simulink model "PlanarModelandControl"  (R2026a) (continued)
== system PlanarModelandControl/Planta/Dinamica do Veículo/Pacejka/Pacejka Long RR == (continued)
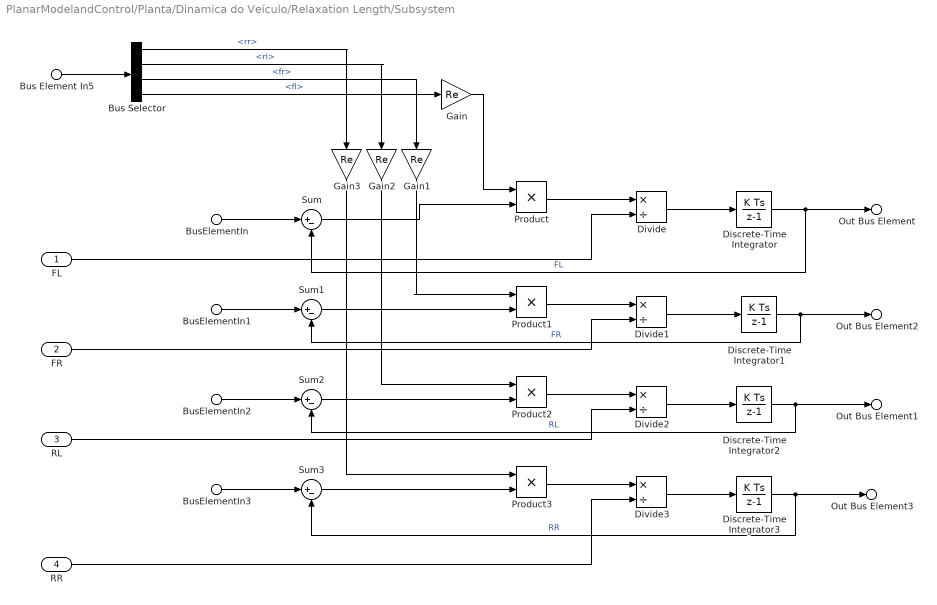
== system PlanarModelandControl/Planta/Dinamica do Veículo/Relaxation Length/Subsystem ==
  FL : Inport
  FR : Inport  Port=2
  RL : Inport  Port=3
  RR : Inport  Port=4
  BusElementIn : Inport  Port=5  PortName=SlipAnges  Element=SA_FL
  BusElementIn1 : Inport  Port=5  PortName=SlipAnges  Element=SA_FR
  BusElementIn2 : Inport  Port=5  PortName=SlipAnges  Element=SA_RL
  BusElementIn3 : Inport  Port=5  PortName=SlipAnges  Element=SA_RR
  Bus Element In5 : Inport  Port=6  PortName=omega
  Bus Selector : BusSelector  OutputSignals=rr,rl,fr,fl
  Discrete-Time Integrator{,1..3} (4 alike) : DiscreteIntegrator  SampleTime=-1  ICPrevOutput=DiscIntNeverNeededParam  ICPrevScaledInput=DiscIntNeverNeededParam
  Divide{,1..3} (4 alike) : Product  Inputs=*/
  Gain{,1..3} (4 alike) : Gain  Gain=Re
  Product{,1..3} (4 alike) : Product
  Sum{,1..3} (4 alike) : Sum  Inputs=|+-
  Out Bus Element : Outport  PortName=RelaxionLength  Element=SAs_FL
  Out Bus Element3 : Outport  PortName=RelaxionLength  Element=SAs_RR
  Out Bus Element2 : Outport  PortName=RelaxionLength  Element=SAs_FR
  Out Bus Element1 : Outport  PortName=RelaxionLength  Element=SAs_RL
  wires:
  BusElementIn/1 -> Sum/1
  BusElementIn1/1 -> Sum1/1
  BusElementIn2/1 -> Sum2/1
  BusElementIn3/1 -> Sum3/1
  Bus Element In5/1 -> Bus Selector/1
  Bus Selector/1 -> Gain3/1  "<rr>"
  Bus Selector/2 -> Gain2/1  "<rl>"
  Bus Selector/3 -> Gain1/1  "<fr>"
  Bus Selector/4 -> Gain/1  "<fl>"
  Sum/1 -> Product/2
  Gain/1 -> Product/1
  Sum3/1 -> Product3/2
  Sum2/1 -> Product2/2
  Sum1/1 -> Product1/2
  Gain1/1 -> Product1/1
  Gain2/1 -> Product2/1
  Gain3/1 -> Product3/1
  Product/1 -> Divide/1
  Product1/1 -> Divide1/1
  Product2/1 -> Divide2/1
  Product3/1 -> Divide3/1
  FL/1 -> Divide/2
  FR/1 -> Divide1/2
  RL/1 -> Divide2/2
  RR/1 -> Divide3/2
  Divide/1 -> Discrete-Time Integrator/1
  Discrete-Time Integrator/1 -> Out Bus Element/1, Sum/2  "FL"
  Discrete-Time Integrator1/1 -> Out Bus Element2/1, Sum1/2  "FR"
  Divide2/1 -> Discrete-Time Integrator2/1
  Divide1/1 -> Discrete-Time Integrator1/1
  Discrete-Time Integrator2/1 -> Out Bus Element1/1, Sum2/2  "RL"
  Discrete-Time Integrator3/1 -> Out Bus Element3/1, Sum3/2  "RR"
  Divide3/1 -> Discrete-Time Integrator3/1

== system PlanarModelandControl/Planta/Dinamica do Veículo/Dinamica da Roda/Slip Ratio/Velocity Margin ==
  Bus Element In1 : Inport  PortName=Kx_FL
  Constant : Constant  Value=Ts
  Gain : Gain  Gain=0.5
  Gain1 : Gain  Gain=(R^2/Iw_+1/M)
  Product : Product
  Output : Outport
  wires:
  Constant/1 -> Gain/1
  Gain1/1 -> Product/2
  Product/1 -> Output/1
  Bus Element In1/1 -> Gain1/1
  Gain/1 -> Product/1

== system PlanarModelandControl/Planta/Dinamica do Veículo/Dinamica da Roda/Slip Ratio/Velocity Margin1 ==
  Bus Element In1 : Inport  PortName=Kx_FR
  Constant : Constant  Value=Ts
  Gain : Gain  Gain=0.5
  Gain1 : Gain  Gain=(R^2/Iw_+1/M)
  Product : Product
  Output : Outport
  wires:
  Product/1 -> Output/1
  Gain1/1 -> Product/2
  Bus Element In1/1 -> Gain1/1
  Constant/1 -> Gain/1
  Gain/1 -> Product/1

== system PlanarModelandControl/Planta/Dinamica do Veículo/Dinamica da Roda/Slip Ratio/Velocity Margin2 ==
  Bus Element In1 : Inport  PortName=Kx_RL
  Constant : Constant  Value=Ts
  Gain : Gain  Gain=0.5
  Gain1 : Gain  Gain=(R^2/Iw_+1/M)
  Product : Product
  Output : Outport
  wires:
  Constant/1 -> Gain/1
  Bus Element In1/1 -> Gain1/1
  Gain1/1 -> Product/2
  Product/1 -> Output/1
  Gain/1 -> Product/1

== system PlanarModelandControl/Planta/Dinamica do Veículo/Dinamica da Roda/Slip Ratio/Velocity Margin3 ==
  Bus Element In1 : Inport  PortName=Kx_RR
  Constant : Constant  Value=Ts
  Gain : Gain  Gain=0.5
  Gain1 : Gain  Gain=(R^2/Iw_+1/M)
  Product : Product
  Output : Outport
  wires:
  Product/1 -> Output/1
  Gain1/1 -> Product/2
  Constant/1 -> Gain/1
  Bus Element In1/1 -> Gain1/1
  Gain/1 -> Product/1

== system PlanarModelandControl/Planta/Dinamica do Veículo/Pacejka/Pacejka Lat FL1/By ==
  KySA : Inport
  Dy : Inport  Port=2
  Cy : Inport  Port=3
  Constant : Constant  Value=0.0001  SampleTime=0.01
  Divide : Product  Inputs=*/
  Product : Product
  Sum : Sum  Inputs=|++
  By : Outport
  wires:
  Cy/1 -> Product/1
  Sum/1 -> Product/2
  Dy/1 -> Sum/1
  Product/1 -> Divide/2
  KySA/1 -> Divide/1
  Constant/1 -> Sum/2
  Divide/1 -> By/1

== system PlanarModelandControl/Planta/Dinamica do Veículo/Pacejka/Pacejka Lat FL1/Dy ==
  Fz : Inport
  dfzy : Inport  Port=2
  Constant : Constant  Value=dy1  SampleTime=0.01
  Gain : Gain  Gain=dy2
  Gain1 : Gain  Gain=1-dy3*IAY^2
  Gain2 : Gain  Gain=1+py3*dpi+py4*dpi^2
  Gain4 : Gain  Gain=-1
  Product : Product
  Sum : Sum  Inputs=|++
  Dy : Outport
  wires:
  dfzy/1 -> Gain/1
  Gain/1 -> Sum/2
  Constant/1 -> Sum/1
  Sum/1 -> Gain1/1
  Gain1/1 -> Gain2/1
  Product/1 -> Dy/1
  Fz/1 -> Gain4/1
  Gain2/1 -> Product/1
  Gain4/1 -> Product/2

== system PlanarModelandControl/Planta/Dinamica do Veículo/Pacejka/Pacejka Lat FL1/Ey ==
  SAy : Inport
  dfzy : Inport  Port=2
  Constant : Constant  Value=ey1  SampleTime=0.01
  Constant1 : Constant  SampleTime=0.01
  Gain : Gain  Gain=ey2
  Gain1 : Gain  Gain=(1+ey5*IAy^2)-(ey3+ey4*IAy)
  Min : MinMax  Inputs=2
  Product : Product
  Sign : Signum
  Sum : Sum  Inputs=|++
  Ey : Outport
  wires:
  dfzy/1 -> Gain/1
  Gain/1 -> Sum/2
  Constant/1 -> Sum/1
  SAy/1 -> Sign/1
  Sign/1 -> Gain1/1
  Product/1 -> Min/1
  Constant1/1 -> Min/2
  Min/1 -> Ey/1
  Gain1/1 -> Product/2
  Sum/1 -> Product/1

== system PlanarModelandControl/Planta/Dinamica do Veículo/Pacejka/Pacejka Lat FL1/KyIA ==
  Fz : Inport
  dfzy : Inport  Port=2
  Constant : Constant  Value=ky6  SampleTime=0.01
  Gain : Gain  Gain=1+py5*dpi
  Gain1 : Gain  Gain=ky7
  Gain3 : Gain  Gain=-1
  Product : Product
  Sum : Sum  Inputs=|++
  KylA : Outport
  wires:
  Gain1/1 -> Sum/2
  dfzy/1 -> Gain1/1
  Constant/1 -> Sum/1
  Gain/1 -> Product/1
  Sum/1 -> Product/2
  Product/1 -> KylA/1
  Fz/1 -> Gain3/1
  Gain3/1 -> Gain/1

== system PlanarModelandControl/Planta/Dinamica do Veículo/Pacejka/Pacejka Lat FL1/KySA ==
  Fz : Inport
  Abs : Abs  SaturateOnIntegerOverflow=off
  Atan : Trigonometry  Operator=atan
  Constant : Constant  Value=(ky2+ky5*IAy^2)*(1+py2*dpi)*Fz0y  SampleTime=0.01
  Constant1 : Constant  Value=IAy  SampleTime=0.01
  Constant2 : Constant  SampleTime=0.01
  Divide : Product  Inputs=*/
  Gain : Gain  Gain=ky4
  Gain1 : Gain  Gain=ky3
  Gain2 : Gain  Gain=ky1*Fz0y
  Gain3 : Gain  Gain=1+py1*dpi
  Gain4 : Gain  Gain=-1
  Product : Product
  Sin : Trigonometry
  Sum : Sum  Inputs=|+-
  KySA : Outport
  wires:
  Constant/1 -> Divide/2
  Divide/1 -> Atan/1
  Atan/1 -> Gain/1
  Gain/1 -> Sin/1
  Gain3/1 -> Gain2/1
  Gain2/1 -> KySA/1
  Constant2/1 -> Sum/1
  Sum/1 -> Product/2
  Abs/1 -> Gain1/1
  Gain1/1 -> Sum/2
  Constant1/1 -> Abs/1
  Sin/1 -> Product/1
  Product/1 -> Gain3/1
  Fz/1 -> Gain4/1
  Gain4/1 -> Divide/1

== system PlanarModelandControl/Planta/Dinamica do Veículo/Pacejka/Pacejka Lat FL1/SAy ==
  SAs : Inport
  SHy : Inport  Port=2
  Sum : Sum  Inputs=|++
  SAy : Outport
  wires:
  SAs/1 -> Sum/1
  SHy/1 -> Sum/2
  Sum/1 -> SAy/1

== system PlanarModelandControl/Planta/Dinamica do Veículo/Pacejka/Pacejka Lat FL1/SHy ==
  KySA : Inport
  KylA : Inport  Port=2
  SVylA : Inport  Port=3
  dfzy : Inport  Port=4
  Constant : Constant  Value=hy1  SampleTime=0.01
  Divide : Product  Inputs=*/
  Gain : Gain  Gain=hy2
  Gain1 : Gain  Gain=IAy
  Sum : Sum  Inputs=|++
  Sum1 : Sum  Inputs=|++
  Sum2 : Sum  Inputs=|+-
  SHy : Outport
  wires:
  dfzy/1 -> Gain/1
  Gain/1 -> Sum/2
  Constant/1 -> Sum/1
  Sum/1 -> Sum1/1
  KylA/1 -> Gain1/1
  Gain1/1 -> Sum2/1
  SVylA/1 -> Sum2/2
  Sum2/1 -> Divide/1
  KySA/1 -> Divide/2
  Divide/1 -> Sum1/2
  Sum1/1 -> SHy/1

== system PlanarModelandControl/Planta/Dinamica do Veículo/Pacejka/Pacejka Lat FL1/SVy ==
  Fz : Inport
  dfzy : Inport  Port=2
  SVylA : Inport  Port=3
  Constant : Constant  Value=vy1  SampleTime=0.01
  Gain : Gain  Gain=vy2
  Gain1 : Gain  Gain=0
  Gain3 : Gain  Gain=-1
  Product : Product
  Sum : Sum  Inputs=|++
  Sum1 : Sum  Inputs=|++
  SVy : Outport
  wires:
  Constant/1 -> Sum/1
  Gain/1 -> Sum/2
  dfzy/1 -> Gain/1
  Sum/1 -> Product/1
  Product/1 -> Sum1/1
  Sum1/1 -> Gain1/1
  SVylA/1 -> Sum1/2
  Fz/1 -> Gain3/1
  Gain1/1 -> SVy/1
  Gain3/1 -> Product/2

== system PlanarModelandControl/Planta/Dinamica do Veículo/Pacejka/Pacejka Lat FL1/SVyIA ==
  Fz : Inport
  dfzy : Inport  Port=2
  Constant : Constant  Value=vy3  SampleTime=0.01
  Gain : Gain  Gain=vy4
  Gain1 : Gain  Gain=IAy
  Gain3 : Gain  Gain=-1
  Product : Product
  Sum : Sum  Inputs=|++
  SVylA : Outport
  wires:
  Constant/1 -> Sum/1
  dfzy/1 -> Gain/1
  Gain/1 -> Sum/2
  Sum/1 -> Gain1/1
  Gain1/1 -> Product/1
  Product/1 -> SVylA/1
  Fz/1 -> Gain3/1
  Gain3/1 -> Product/2

== system PlanarModelandControl/Planta/Dinamica do Veículo/Pacejka/Pacejka Lat FL1/dfzy ==
  Fz : Inport
  Constant : Constant  Value=Fz0y  SampleTime=0.01
  Divide : Product  Inputs=*/
  Gain3 : Gain  Gain=-1
  Sum : Sum  Inputs=|+-
  dfzy : Outport
  wires:
  Divide/1 -> dfzy/1
  Sum/1 -> Divide/1
  Constant/1 -> Sum/2, Divide/2
  Fz/1 -> Gain3/1
  Gain3/1 -> Sum/1

== system PlanarModelandControl/Planta/Dinamica do Veículo/Pacejka/Pacejka Lat FL2/By ==
  KySA : Inport
  Dy : Inport  Port=2
  Cy : Inport  Port=3
  Constant : Constant  Value=0.0001  SampleTime=0.01
  Divide : Product  Inputs=*/
  Product : Product
  Sum : Sum  Inputs=|++
  By : Outport
  wires:
  Cy/1 -> Product/1
  Sum/1 -> Product/2
  Dy/1 -> Sum/1
  Product/1 -> Divide/2
  KySA/1 -> Divide/1
  Constant/1 -> Sum/2
  Divide/1 -> By/1

== system PlanarModelandControl/Planta/Dinamica do Veículo/Pacejka/Pacejka Lat FL2/Dy ==
  Fz : Inport
  dfzy : Inport  Port=2
  Constant : Constant  Value=dy1  SampleTime=0.01
  Gain : Gain  Gain=dy2
  Gain1 : Gain  Gain=1-dy3*IAY^2
  Gain2 : Gain  Gain=1+py3*dpi+py4*dpi^2
  Gain4 : Gain  Gain=-1
  Product : Product
  Sum : Sum  Inputs=|++
  Dy : Outport
  wires:
  dfzy/1 -> Gain/1
  Gain/1 -> Sum/2
  Constant/1 -> Sum/1
  Sum/1 -> Gain1/1
  Gain1/1 -> Gain2/1
  Product/1 -> Dy/1
  Fz/1 -> Gain4/1
  Gain2/1 -> Product/1
  Gain4/1 -> Product/2

== system PlanarModelandControl/Planta/Dinamica do Veículo/Pacejka/Pacejka Lat FL2/Ey ==
  SAy : Inport
  dfzy : Inport  Port=2
  Constant : Constant  Value=ey1  SampleTime=0.01
  Constant1 : Constant  SampleTime=0.01
  Gain : Gain  Gain=ey2
  Gain1 : Gain  Gain=(1+ey5*IAy^2)-(ey3+ey4*IAy)
  Min : MinMax  Inputs=2
  Product : Product
  Sign : Signum
  Sum : Sum  Inputs=|++
  Ey : Outport
  wires:
  dfzy/1 -> Gain/1
  Gain/1 -> Sum/2
  Constant/1 -> Sum/1
  SAy/1 -> Sign/1
  Sign/1 -> Gain1/1
  Product/1 -> Min/1
  Constant1/1 -> Min/2
  Min/1 -> Ey/1
  Gain1/1 -> Product/2
  Sum/1 -> Product/1

== system PlanarModelandControl/Planta/Dinamica do Veículo/Pacejka/Pacejka Lat FL2/KyIA ==
  Fz : Inport
  dfzy : Inport  Port=2
  Constant : Constant  Value=ky6  SampleTime=0.01
  Gain : Gain  Gain=1+py5*dpi
  Gain1 : Gain  Gain=ky7
  Gain3 : Gain  Gain=-1
  Product : Product
  Sum : Sum  Inputs=|++
  KylA : Outport
  wires:
  Gain1/1 -> Sum/2
  dfzy/1 -> Gain1/1
  Constant/1 -> Sum/1
  Gain/1 -> Product/1
  Sum/1 -> Product/2
  Product/1 -> KylA/1
  Fz/1 -> Gain3/1
  Gain3/1 -> Gain/1

== system PlanarModelandControl/Planta/Dinamica do Veículo/Pacejka/Pacejka Lat FL2/KySA ==
  Fz : Inport
  Abs : Abs  SaturateOnIntegerOverflow=off
  Atan : Trigonometry  Operator=atan
  Constant : Constant  Value=(ky2+ky5*IAy^2)*(1+py2*dpi)*Fz0y  SampleTime=0.01
  Constant1 : Constant  Value=IAy  SampleTime=0.01
  Constant2 : Constant  SampleTime=0.01
  Divide : Product  Inputs=*/
  Gain : Gain  Gain=ky4
  Gain1 : Gain  Gain=ky3
  Gain2 : Gain  Gain=ky1*Fz0y
  Gain3 : Gain  Gain=1+py1*dpi
  Gain4 : Gain  Gain=-1
  Product : Product
  Sin : Trigonometry
  Sum : Sum  Inputs=|+-
  KySA : Outport
  wires:
  Constant/1 -> Divide/2
  Divide/1 -> Atan/1
  Atan/1 -> Gain/1
  Gain/1 -> Sin/1
  Gain3/1 -> Gain2/1
  Gain2/1 -> KySA/1
  Constant2/1 -> Sum/1
  Sum/1 -> Product/2
  Abs/1 -> Gain1/1
  Gain1/1 -> Sum/2
  Constant1/1 -> Abs/1
  Sin/1 -> Product/1
  Product/1 -> Gain3/1
  Fz/1 -> Gain4/1
  Gain4/1 -> Divide/1

== system PlanarModelandControl/Planta/Dinamica do Veículo/Pacejka/Pacejka Lat FL2/SAy ==
  SAs : Inport
  SHy : Inport  Port=2
  Sum : Sum  Inputs=|++
  SAy : Outport
  wires:
  SAs/1 -> Sum/1
  SHy/1 -> Sum/2
  Sum/1 -> SAy/1

== system PlanarModelandControl/Planta/Dinamica do Veículo/Pacejka/Pacejka Lat FL2/SHy ==
  KySA : Inport
  KylA : Inport  Port=2
  SVylA : Inport  Port=3
  dfzy : Inport  Port=4
  Constant : Constant  Value=hy1  SampleTime=0.01
  Divide : Product  Inputs=*/
  Gain : Gain  Gain=hy2
  Gain1 : Gain  Gain=IAy
  Sum : Sum  Inputs=|++
  Sum1 : Sum  Inputs=|++
  Sum2 : Sum  Inputs=|+-
  SHy : Outport
  wires:
  dfzy/1 -> Gain/1
  Gain/1 -> Sum/2
  Constant/1 -> Sum/1
  Sum/1 -> Sum1/1
  KylA/1 -> Gain1/1
  Gain1/1 -> Sum2/1
  SVylA/1 -> Sum2/2
  Sum2/1 -> Divide/1
  KySA/1 -> Divide/2
  Divide/1 -> Sum1/2
  Sum1/1 -> SHy/1

== system PlanarModelandControl/Planta/Dinamica do Veículo/Pacejka/Pacejka Lat FL2/SVy ==
  Fz : Inport
  dfzy : Inport  Port=2
  SVylA : Inport  Port=3
  Constant : Constant  Value=vy1  SampleTime=0.01
  Gain : Gain  Gain=vy2
  Gain1 : Gain  Gain=0
  Gain3 : Gain  Gain=-1
  Product : Product
  Sum : Sum  Inputs=|++
  Sum1 : Sum  Inputs=|++
  SVy : Outport
  wires:
  Constant/1 -> Sum/1
  Gain/1 -> Sum/2
  dfzy/1 -> Gain/1
  Sum/1 -> Product/1
  Product/1 -> Sum1/1
  Sum1/1 -> Gain1/1
  SVylA/1 -> Sum1/2
  Fz/1 -> Gain3/1
  Gain1/1 -> SVy/1
  Gain3/1 -> Product/2

== system PlanarModelandControl/Planta/Dinamica do Veículo/Pacejka/Pacejka Lat FL2/SVyIA ==
  Fz : Inport
  dfzy : Inport  Port=2
  Constant : Constant  Value=vy3  SampleTime=0.01
  Gain : Gain  Gain=vy4
  Gain1 : Gain  Gain=IAy
  Gain3 : Gain  Gain=-1
  Product : Product
  Sum : Sum  Inputs=|++
  SVylA : Outport
  wires:
  Constant/1 -> Sum/1
  dfzy/1 -> Gain/1
  Gain/1 -> Sum/2
  Sum/1 -> Gain1/1
  Gain1/1 -> Product/1
  Product/1 -> SVylA/1
  Fz/1 -> Gain3/1
  Gain3/1 -> Product/2

== system PlanarModelandControl/Planta/Dinamica do Veículo/Pacejka/Pacejka Lat FL2/dfzy ==
  Fz : Inport
  Constant : Constant  Value=Fz0y  SampleTime=0.01
  Divide : Product  Inputs=*/
  Gain3 : Gain  Gain=-1
  Sum : Sum  Inputs=|+-
  dfzy : Outport
  wires:
  Divide/1 -> dfzy/1
  Sum/1 -> Divide/1
  Constant/1 -> Sum/2, Divide/2
  Fz/1 -> Gain3/1
  Gain3/1 -> Sum/1

== system PlanarModelandControl/Planta/Dinamica do Veículo/Pacejka/Pacejka Lat FL3/By ==
  KySA : Inport
  Dy : Inport  Port=2
  Cy : Inport  Port=3
  Constant : Constant  Value=0.0001  SampleTime=0.01
  Divide : Product  Inputs=*/
  Product : Product
  Sum : Sum  Inputs=|++
  By : Outport
  wires:
  Cy/1 -> Product/1
  Sum/1 -> Product/2
  Dy/1 -> Sum/1
  Product/1 -> Divide/2
  KySA/1 -> Divide/1
  Constant/1 -> Sum/2
  Divide/1 -> By/1

== system PlanarModelandControl/Planta/Dinamica do Veículo/Pacejka/Pacejka Lat FL3/Dy ==
  Fz : Inport
  dfzy : Inport  Port=2
  Constant : Constant  Value=dy1  SampleTime=0.01
  Gain : Gain  Gain=dy2
  Gain1 : Gain  Gain=1-dy3*IAY^2
  Gain2 : Gain  Gain=1+py3*dpi+py4*dpi^2
  Gain4 : Gain  Gain=-1
  Product : Product
  Sum : Sum  Inputs=|++
  Dy : Outport
  wires:
  dfzy/1 -> Gain/1
  Gain/1 -> Sum/2
  Constant/1 -> Sum/1
  Sum/1 -> Gain1/1
  Gain1/1 -> Gain2/1
  Product/1 -> Dy/1
  Fz/1 -> Gain4/1
  Gain2/1 -> Product/1
  Gain4/1 -> Product/2

== system PlanarModelandControl/Planta/Dinamica do Veículo/Pacejka/Pacejka Lat FL3/Ey ==
  SAy : Inport
  dfzy : Inport  Port=2
  Constant : Constant  Value=ey1  SampleTime=0.01
  Constant1 : Constant  SampleTime=0.01
  Gain : Gain  Gain=ey2
  Gain1 : Gain  Gain=(1+ey5*IAy^2)-(ey3+ey4*IAy)
  Min : MinMax  Inputs=2
  Product : Product
  Sign : Signum
  Sum : Sum  Inputs=|++
  Ey : Outport
  wires:
  dfzy/1 -> Gain/1
  Gain/1 -> Sum/2
  Constant/1 -> Sum/1
  SAy/1 -> Sign/1
  Sign/1 -> Gain1/1
  Product/1 -> Min/1
  Constant1/1 -> Min/2
  Min/1 -> Ey/1
  Gain1/1 -> Product/2
  Sum/1 -> Product/1

== system PlanarModelandControl/Planta/Dinamica do Veículo/Pacejka/Pacejka Lat FL3/KyIA ==
  Fz : Inport
  dfzy : Inport  Port=2
  Constant : Constant  Value=ky6  SampleTime=0.01
  Gain : Gain  Gain=1+py5*dpi
  Gain1 : Gain  Gain=ky7
  Gain3 : Gain  Gain=-1
  Product : Product
  Sum : Sum  Inputs=|++
  KylA : Outport
  wires:
  Gain1/1 -> Sum/2
  dfzy/1 -> Gain1/1
  Constant/1 -> Sum/1
  Gain/1 -> Product/1
  Sum/1 -> Product/2
  Product/1 -> KylA/1
  Fz/1 -> Gain3/1
  Gain3/1 -> Gain/1

== system PlanarModelandControl/Planta/Dinamica do Veículo/Pacejka/Pacejka Lat FL3/KySA ==
  Fz : Inport
  Abs : Abs  SaturateOnIntegerOverflow=off
  Atan : Trigonometry  Operator=atan
  Constant : Constant  Value=(ky2+ky5*IAy^2)*(1+py2*dpi)*Fz0y  SampleTime=0.01
  Constant1 : Constant  Value=IAy  SampleTime=0.01
  Constant2 : Constant  SampleTime=0.01
  Divide : Product  Inputs=*/
  Gain : Gain  Gain=ky4
  Gain1 : Gain  Gain=ky3
  Gain2 : Gain  Gain=ky1*Fz0y
  Gain3 : Gain  Gain=1+py1*dpi
  Gain4 : Gain  Gain=-1
  Product : Product
  Sin : Trigonometry
  Sum : Sum  Inputs=|+-
  KySA : Outport
  wires:
  Constant/1 -> Divide/2
  Divide/1 -> Atan/1
  Atan/1 -> Gain/1
  Gain/1 -> Sin/1
  Gain3/1 -> Gain2/1
  Gain2/1 -> KySA/1
  Constant2/1 -> Sum/1
  Sum/1 -> Product/2
  Abs/1 -> Gain1/1
  Gain1/1 -> Sum/2
  Constant1/1 -> Abs/1
  Sin/1 -> Product/1
  Product/1 -> Gain3/1
  Fz/1 -> Gain4/1
  Gain4/1 -> Divide/1

== system PlanarModelandControl/Planta/Dinamica do Veículo/Pacejka/Pacejka Lat FL3/SAy ==
  SAs : Inport
  SHy : Inport  Port=2
  Sum : Sum  Inputs=|++
  SAy : Outport
  wires:
  SAs/1 -> Sum/1
  SHy/1 -> Sum/2
  Sum/1 -> SAy/1

== system PlanarModelandControl/Planta/Dinamica do Veículo/Pacejka/Pacejka Lat FL3/SHy ==
  KySA : Inport
  KylA : Inport  Port=2
  SVylA : Inport  Port=3
  dfzy : Inport  Port=4
  Constant : Constant  Value=hy1  SampleTime=0.01
  Divide : Product  Inputs=*/
  Gain : Gain  Gain=hy2
  Gain1 : Gain  Gain=IAy
  Sum : Sum  Inputs=|++
  Sum1 : Sum  Inputs=|++
  Sum2 : Sum  Inputs=|+-
  SHy : Outport
  wires:
  dfzy/1 -> Gain/1
  Gain/1 -> Sum/2
  Constant/1 -> Sum/1
  Sum/1 -> Sum1/1
  KylA/1 -> Gain1/1
  Gain1/1 -> Sum2/1
  SVylA/1 -> Sum2/2
  Sum2/1 -> Divide/1
  KySA/1 -> Divide/2
  Divide/1 -> Sum1/2
  Sum1/1 -> SHy/1

== system PlanarModelandControl/Planta/Dinamica do Veículo/Pacejka/Pacejka Lat FL3/SVy ==
  Fz : Inport
  dfzy : Inport  Port=2
  SVylA : Inport  Port=3
  Constant : Constant  Value=vy1  SampleTime=0.01
  Gain : Gain  Gain=vy2
  Gain1 : Gain  Gain=0
  Gain3 : Gain  Gain=-1
  Product : Product
  Sum : Sum  Inputs=|++
  Sum1 : Sum  Inputs=|++
  SVy : Outport
  wires:
  Constant/1 -> Sum/1
  Gain/1 -> Sum/2
  dfzy/1 -> Gain/1
  Sum/1 -> Product/1
  Product/1 -> Sum1/1
  Sum1/1 -> Gain1/1
  SVylA/1 -> Sum1/2
  Fz/1 -> Gain3/1
  Gain1/1 -> SVy/1
  Gain3/1 -> Product/2

== system PlanarModelandControl/Planta/Dinamica do Veículo/Pacejka/Pacejka Lat FL3/SVyIA ==
  Fz : Inport
  dfzy : Inport  Port=2
  Constant : Constant  Value=vy3  SampleTime=0.01
  Gain : Gain  Gain=vy4
  Gain1 : Gain  Gain=IAy
  Gain3 : Gain  Gain=-1
  Product : Product
  Sum : Sum  Inputs=|++
  SVylA : Outport
  wires:
  Constant/1 -> Sum/1
  dfzy/1 -> Gain/1
  Gain/1 -> Sum/2
  Sum/1 -> Gain1/1
  Gain1/1 -> Product/1
  Product/1 -> SVylA/1
  Fz/1 -> Gain3/1
  Gain3/1 -> Product/2

== system PlanarModelandControl/Planta/Dinamica do Veículo/Pacejka/Pacejka Lat FL3/dfzy ==
  Fz : Inport
  Constant : Constant  Value=Fz0y  SampleTime=0.01
  Divide : Product  Inputs=*/
  Gain3 : Gain  Gain=-1
  Sum : Sum  Inputs=|+-
  dfzy : Outport
  wires:
  Divide/1 -> dfzy/1
  Sum/1 -> Divide/1
  Constant/1 -> Sum/2, Divide/2
  Fz/1 -> Gain3/1
  Gain3/1 -> Sum/1

== system PlanarModelandControl/Planta/Dinamica do Veículo/Pacejka/Pacejka Lat FL4/By ==
  KySA : Inport
  Dy : Inport  Port=2
  Cy : Inport  Port=3
  Constant : Constant  Value=0.0001  SampleTime=0.01
  Divide : Product  Inputs=*/
  Product : Product
  Sum : Sum  Inputs=|++
  By : Outport
  wires:
  Cy/1 -> Product/1
  Sum/1 -> Product/2
  Dy/1 -> Sum/1
  Product/1 -> Divide/2
  KySA/1 -> Divide/1
  Constant/1 -> Sum/2
  Divide/1 -> By/1

== system PlanarModelandControl/Planta/Dinamica do Veículo/Pacejka/Pacejka Lat FL4/Dy ==
  Fz : Inport
  dfzy : Inport  Port=2
  Constant : Constant  Value=dy1  SampleTime=0.01
  Gain : Gain  Gain=dy2
  Gain1 : Gain  Gain=1-dy3*IAY^2
  Gain2 : Gain  Gain=1+py3*dpi+py4*dpi^2
  Gain4 : Gain  Gain=-1
  Product : Product
  Sum : Sum  Inputs=|++
  Dy : Outport
  wires:
  dfzy/1 -> Gain/1
  Gain/1 -> Sum/2
  Constant/1 -> Sum/1
  Sum/1 -> Gain1/1
  Gain1/1 -> Gain2/1
  Product/1 -> Dy/1
  Fz/1 -> Gain4/1
  Gain2/1 -> Product/1
  Gain4/1 -> Product/2

== system PlanarModelandControl/Planta/Dinamica do Veículo/Pacejka/Pacejka Lat FL4/Ey ==
  SAy : Inport
  dfzy : Inport  Port=2
  Constant : Constant  Value=ey1  SampleTime=0.01
  Constant1 : Constant  SampleTime=0.01
  Gain : Gain  Gain=ey2
  Gain1 : Gain  Gain=(1+ey5*IAy^2)-(ey3+ey4*IAy)
  Min : MinMax  Inputs=2
  Product : Product
  Sign : Signum
  Sum : Sum  Inputs=|++
  Ey : Outport
  wires:
  dfzy/1 -> Gain/1
  Gain/1 -> Sum/2
  Constant/1 -> Sum/1
  SAy/1 -> Sign/1
  Sign/1 -> Gain1/1
  Product/1 -> Min/1
  Constant1/1 -> Min/2
  Min/1 -> Ey/1
  Gain1/1 -> Product/2
  Sum/1 -> Product/1

== system PlanarModelandControl/Planta/Dinamica do Veículo/Pacejka/Pacejka Lat FL4/KyIA ==
  Fz : Inport
  dfzy : Inport  Port=2
  Constant : Constant  Value=ky6  SampleTime=0.01
  Gain : Gain  Gain=1+py5*dpi
  Gain1 : Gain  Gain=ky7
  Gain3 : Gain  Gain=-1
  Product : Product
  Sum : Sum  Inputs=|++
  KylA : Outport
  wires:
  Gain1/1 -> Sum/2
  dfzy/1 -> Gain1/1
  Constant/1 -> Sum/1
  Gain/1 -> Product/1
  Sum/1 -> Product/2
  Product/1 -> KylA/1
  Fz/1 -> Gain3/1
  Gain3/1 -> Gain/1

== system PlanarModelandControl/Planta/Dinamica do Veículo/Pacejka/Pacejka Lat FL4/KySA ==
  Fz : Inport
  Abs : Abs  SaturateOnIntegerOverflow=off
  Atan : Trigonometry  Operator=atan
  Constant : Constant  Value=(ky2+ky5*IAy^2)*(1+py2*dpi)*Fz0y  SampleTime=0.01
  Constant1 : Constant  Value=IAy  SampleTime=0.01
  Constant2 : Constant  SampleTime=0.01
  Divide : Product  Inputs=*/
  Gain : Gain  Gain=ky4
  Gain1 : Gain  Gain=ky3
  Gain2 : Gain  Gain=ky1*Fz0y
  Gain3 : Gain  Gain=1+py1*dpi
  Gain4 : Gain  Gain=-1
  Product : Product
  Sin : Trigonometry
  Sum : Sum  Inputs=|+-
  KySA : Outport
  wires:
  Constant/1 -> Divide/2
  Divide/1 -> Atan/1
  Atan/1 -> Gain/1
  Gain/1 -> Sin/1
  Gain3/1 -> Gain2/1
  Gain2/1 -> KySA/1
  Constant2/1 -> Sum/1
  Sum/1 -> Product/2
  Abs/1 -> Gain1/1
  Gain1/1 -> Sum/2
  Constant1/1 -> Abs/1
  Sin/1 -> Product/1
  Product/1 -> Gain3/1
  Fz/1 -> Gain4/1
  Gain4/1 -> Divide/1

== system PlanarModelandControl/Planta/Dinamica do Veículo/Pacejka/Pacejka Lat FL4/SAy ==
  SAs : Inport
  SHy : Inport  Port=2
  Sum : Sum  Inputs=|++
  SAy : Outport
  wires:
  SAs/1 -> Sum/1
  SHy/1 -> Sum/2
  Sum/1 -> SAy/1

== system PlanarModelandControl/Planta/Dinamica do Veículo/Pacejka/Pacejka Lat FL4/SHy ==
  KySA : Inport
  KylA : Inport  Port=2
  SVylA : Inport  Port=3
  dfzy : Inport  Port=4
  Constant : Constant  Value=hy1  SampleTime=0.01
  Divide : Product  Inputs=*/
  Gain : Gain  Gain=hy2
  Gain1 : Gain  Gain=IAy
  Sum : Sum  Inputs=|++
  Sum1 : Sum  Inputs=|++
  Sum2 : Sum  Inputs=|+-
  SHy : Outport
  wires:
  dfzy/1 -> Gain/1
  Gain/1 -> Sum/2
  Constant/1 -> Sum/1
  Sum/1 -> Sum1/1
  KylA/1 -> Gain1/1
  Gain1/1 -> Sum2/1
  SVylA/1 -> Sum2/2
  Sum2/1 -> Divide/1
  KySA/1 -> Divide/2
  Divide/1 -> Sum1/2
  Sum1/1 -> SHy/1

== system PlanarModelandControl/Planta/Dinamica do Veículo/Pacejka/Pacejka Lat FL4/SVy ==
  Fz : Inport
  dfzy : Inport  Port=2
  SVylA : Inport  Port=3
  Constant : Constant  Value=vy1  SampleTime=0.01
  Gain : Gain  Gain=vy2
  Gain1 : Gain  Gain=0
  Gain3 : Gain  Gain=-1
  Product : Product
  Sum : Sum  Inputs=|++
  Sum1 : Sum  Inputs=|++
  SVy : Outport
  wires:
  Constant/1 -> Sum/1
  Gain/1 -> Sum/2
  dfzy/1 -> Gain/1
  Sum/1 -> Product/1
  Product/1 -> Sum1/1
  Sum1/1 -> Gain1/1
  SVylA/1 -> Sum1/2
  Fz/1 -> Gain3/1
  Gain1/1 -> SVy/1
  Gain3/1 -> Product/2

== system PlanarModelandControl/Planta/Dinamica do Veículo/Pacejka/Pacejka Lat FL4/SVyIA ==
  Fz : Inport
  dfzy : Inport  Port=2
  Constant : Constant  Value=vy3  SampleTime=0.01
  Gain : Gain  Gain=vy4
  Gain1 : Gain  Gain=IAy
  Gain3 : Gain  Gain=-1
  Product : Product
  Sum : Sum  Inputs=|++
  SVylA : Outport
  wires:
  Constant/1 -> Sum/1
  dfzy/1 -> Gain/1
  Gain/1 -> Sum/2
  Sum/1 -> Gain1/1
  Gain1/1 -> Product/1
  Product/1 -> SVylA/1
  Fz/1 -> Gain3/1
  Gain3/1 -> Product/2

== system PlanarModelandControl/Planta/Dinamica do Veículo/Pacejka/Pacejka Lat FL4/dfzy ==
  Fz : Inport
  Constant : Constant  Value=Fz0y  SampleTime=0.01
  Divide : Product  Inputs=*/
  Gain3 : Gain  Gain=-1
  Sum : Sum  Inputs=|+-
  dfzy : Outport
  wires:
  Divide/1 -> dfzy/1
  Sum/1 -> Divide/1
  Constant/1 -> Sum/2, Divide/2
  Fz/1 -> Gain3/1
  Gain3/1 -> Sum/1

== system PlanarModelandControl/Planta/Dinamica do Veículo/Pacejka/Pacejka Long FL /Bx ==
  Kx : Inport
  Dx : Inport  Port=2
  Divide : Product  Inputs=*/
  Gain : Gain  Gain=cx1
  Bx : Outport
  wires:
  Kx/1 -> Divide/1
  Dx/1 -> Gain/1
  Gain/1 -> Divide/2
  Divide/1 -> Bx/1
